simulink model "ofdm_txrx_supermimo_coded"  (Simulink 7.1)
settings: Solver=VariableStepDiscrete  SolverMode=SingleTasking  StartTime=0.0  StopTime=2*simOnly_simLength  RelTol=1e-3
PostLoadFcn:
  | ofdm_rx_supermimo_init
InitFcn:
  | ofdm_rx_supermimo_init
StartFcn:
  | ofdm_rx_supermimo_init
values from neighbouring files (symbols this model uses):
  Action0_Config = 0* 31*2^18 + 0*2^24 + PHY_AUTORESPONSE_REQ_GOODHDR + PHY_AUTORESPONSE_REQ_GOODPKT + PHY_AUTORESPONSE_REQ_FLAGA    [ofdm_tx_supermimo_init.m]
  Action1_Config = 0* 62*2^18 + PHY_AUTORESPONSE_REQ_GOODHDR + PHY_AUTORESPONSE_REQ_GOODPKT    [ofdm_tx_supermimo_init.m]
  Action2_Config = 0    [ofdm_tx_supermimo_init.m]
  Action3_Config = 0    [ofdm_tx_supermimo_init.m]
  Action4_Config = 0    [ofdm_tx_supermimo_init.m]
  Action5_Config = 0    [ofdm_tx_supermimo_init.m]
  ActionID_AFTransmit = 31    [ofdm_tx_supermimo_init.m]
  ActionID_Disabled = 0    [ofdm_tx_supermimo_init.m]
  ActionID_SetFlagA = 62    [ofdm_tx_supermimo_init.m]
  ActionID_SetFlagB = 61    [ofdm_tx_supermimo_init.m]
  BER_RAM_init_size = 2048    [ofdm_tx_supermimo_init.m]
  channel_h = [1 0; 1 0] * 0.3    [ofdm_tx_supermimo_init.m]
  CPLength = 16    [ofdm_tx_supermimo_init.m]
  FEC_Config = fec_coding_en*1 + fec_soft_dec*2 + fec_qpsk_scl*2^4 + fec_16qam_scl*2^8    (= 4195)    [ofdm_tx_supermimo_init.m]
  longCorr_coef_i = [-4*real(LTS) 0]    [ofdm_rx_supermimo_init.m]
  longCorr_coef_q = [4*imag(LTS) 0]    [ofdm_rx_supermimo_init.m]
  Match0_Config = 0    [ofdm_tx_supermimo_init.m]
  Match1_Config = 0    [ofdm_tx_supermimo_init.m]
  Match2_Config = 0    [ofdm_tx_supermimo_init.m]
  Match3_Config = 0    [ofdm_tx_supermimo_init.m]
  Match4_Config = 0    [ofdm_tx_supermimo_init.m]
  Match5_Config = 0    [ofdm_tx_supermimo_init.m]
  Match6_Config = 0    [ofdm_tx_supermimo_init.m]
  Match7_Config = 0    [ofdm_tx_supermimo_init.m]
  max_CP_length = 16    [ofdm_tx_supermimo_init.m]
  max_num_baseRateSymbols = 31    [ofdm_tx_supermimo_init.m]
  max_num_subcarriers = 64    [ofdm_tx_supermimo_init.m]
  max_num_trainingSymbols = 15    [ofdm_tx_supermimo_init.m]
  max_numBytes = 16384    [ofdm_tx_supermimo_init.m]
  max_OFDM_symbols = 1023    [ofdm_tx_supermimo_init.m]
  modConstellation_qam16 = .75*[1 3 -1 -3]./3    [ofdm_tx_supermimo_init.m]
  modConstellation_qam64 = 0.875*[3 1 5 7 -3 -1 -5 -7]./7    [ofdm_tx_supermimo_init.m]
  modConstellation_qpsk = [1 -1]./sqrt(2)    [ofdm_tx_supermimo_init.m]
  numBaseRateSymbols = numBaseRateSymbols *2    [ofdm_tx_supermimo_init.m]
  numSubcarriers = 64    [ofdm_tx_supermimo_init.m]
  numTrainingSymbols = 2    [ofdm_tx_supermimo_init.m]
  pilot_indicies = 7 + ( (64-21) * 2^8) + ( (64-7) * 2^16) + (21 * 2^24)    (= 356068103)    [ofdm_tx_supermimo_init.m]
  pilotValues = hex2dec('A57D') + (2^16 * hex2dec('5A82'))    [ofdm_tx_supermimo_init.m]
  pktByteNums = numHeaderBytes +  				byteIndex_numPayloadBytes(1)*2^8 +  				byteIndex_numPayloadBytes(2)*2^16 +  				byteIndex_simpleDynModMasks * 2^24    [ofdm_rx_supermimo_init.m]
  PktDet_Delay = 58+32 +      Symbol_Timing_Offset*2^7 +      0*2^16 +      (117* 2^24)    (= 1962935642)    [ofdm_rx_supermimo_init.m]
  pktTiming_count_payloadStart = pktTiming_count_lsStart + pktTiming_ls_num*pktTiming_ls_length    [ofdm_rx_supermimo_init.m]
  preamble = preamble_scale*[shortsyms_10 longsyms_2]    [ofdm_tx_supermimo_init.m]
  preamble_ROM = preamble_I_ROM + preamble_Q_ROM*2^16    [ofdm_tx_supermimo_init.m]
  RAM_init_size = max_numBytes    (= 16384)    [ofdm_tx_supermimo_init.m]
  reg_AF_Tx_Blanking = (320+80) + ( (320+80+79)*2^16)    (= 31392144)    [ofdm_rx_supermimo_init.m]
  reg_AF_Tx_Scaling = 2944    [ofdm_rx_supermimo_init.m]
  reg_Interrupt_PktBuf_Control = reg_InterruptControl +  	1 * 2^16 +  	0 * 2^24    (= 65536)    [ofdm_rx_supermimo_init.m]
  reg_PilotCalcParams = (4 * 2^16) + (minPilotChanMag)    (= 262184.96)    [ofdm_rx_supermimo_init.m]
  regRx_chanEst_minMag = ((0.5)*2^15) + ((0.5 * 2^15)*2^16)    (= 1073758208)    [ofdm_rx_supermimo_init.m]
  regRx_coarseCalcCorrection = round((1.3e-3 - 1.2982e-3)*2^32)    [ofdm_rx_supermimo_init.m]
  regRx_pilotCalcCorrection = round(1.00115 * 2^31)    [ofdm_rx_supermimo_init.m]
  regRx_preCFOoptions = 0 * 2^0 +  	1 * 2^1 +  	0    (= 2)    [ofdm_rx_supermimo_init.m]
  rx_controlBits = 1 * 2  		 + 1 * 4  		 + 0 * 8  		 + 1 * 16 * rx_SISO_Mode  		 + 1 * 32  		 + 1 * 64  		 + 1 * 128  		 + 1 * 256  		 + 1 * 512  		 + 1 * 1024  		 + 1 * 4096  		 + 0 * 16384  		 + 0 * 32768  		 + 1 * 65536  		 + 0 * 131072  		 + 0 * 262144  		 + 1 * 524288  		 + 1 * 1048576 * tx_Alamouti_Mode  		 + 0 * 2097152           + 1 * 33554432  		 + 0 * 4194304  		 + 0 * 8388608  		 + 0 * 16777216  		 + 1 *  ...(+49 chars)    [ofdm_rx_supermimo_init.m]
  Rx_PktDet_LongCorr_Params = LongCorrCounterLoad + 0*2^8 + 0*2^16 + 255*2^24    (= 4278190325)    [ofdm_rx_supermimo_init.m]
  Rx_PktDet_LongCorrThresholds = 8000    [ofdm_rx_supermimo_init.m]
  rx_postEq_scaling = round(rxScaling*2^11) * (1 + 2^16)    [ofdm_rx_supermimo_init.m]
  RxDataScrambling_Seq = [40 198 78 63 82 173 102 245 48 111 172 115 147 230 216 93 72 65 62 2 205 242 122 90 128 83 105 97 73 10 5 252]    [ofdm_tx_supermimo_init.m]
  RxReg_FixedPktLen = 0    [ofdm_rx_supermimo_init.m]
  rxReg_pktDetCorr = 2^00 * (floor(0.7*128)) +  	2^08 * (300) +  	0    [ofdm_rx_supermimo_init.m]
  shortSymbol_time = shortSymbol_time(1:16).'    [ofdm_tx_supermimo_init.m]
  simOnly_numSamples = length(preamble)+( (numSubcarriers+CPLength)*(numTrainingSymbols + numBaseRateSymbols + numFullRateSymbols/2) )    [ofdm_tx_supermimo_init.m]
  train = [train train]    [ofdm_tx_supermimo_init.m]
  tx_controlBits = (antB_preambleShift * 2^4) + (2^1 * tx_Alamouti_Mode) +  	(2^0 * tx_SISO_Mode) + 4*0 + 8*1 + 0*256 + 512*0 + 1024*0 +  	0*hex2dec('1000') + 0*hex2dec('2000') + 0*hex2dec('4000') +  	1*hex2dec('8000') + 1*hex2dec('10000') + hex2dec('20000') +  	0*hex2dec('100000')    [ofdm_tx_supermimo_init.m]
  tx_delays = (50*2^0) + (0*2^8) + (30*2^12)    (= 122930)    [ofdm_tx_supermimo_init.m]
  tx_scaling = (3072) + (12288 * 2^16)    (= 805309440)    [ofdm_tx_supermimo_init.m]
  TxDataScrambling_Seq = [40 198 78 63 82 173 102 245 48 111 172 115 147 230 216 93 72 65 62 2 205 242 122 90 128 83 105 97 73 10 5 252]    [ofdm_tx_supermimo_init.m]
  txReg_symbolCounts = (2^8 *numBaseRateSymbols ) + numTrainingSymbols    [ofdm_tx_supermimo_init.m]
  TxRx_FFTScaling = (3 * 2^0) +  	(2 * 2^2) +  	(1 * 2^4) +  	(1 * 2^6) +  	(1 * 2^8) +  	(0 * 2^10)    (= 347)    [ofdm_tx_supermimo_init.m]
undefined symbols (not defined in the file or in its folder): AntA, AntB, BaseRate, Down, gcbh, numBuffs, numBytes, Up, xlBlockLoadCallback, xlGetSimulinkPeriod, xlipgetpartsetting
libraries: xbsIndex_r4 x6000, simulink x4, dspsigops x4, commchan3 x2, dspsrcs4 x2, commrflib2 x2
(part 13 of 63)

== system ofdm_txrx_supermimo_coded/OFDM Rx MIMO/Coarse Freq Correction/A*B ==
  Re[A] : Inport  OutDataType=sfix(16)  OutScaling=2^0
  Im[A] : Inport  Port=2  OutDataType=sfix(16)  OutScaling=2^0
  Im[B] : Inport  Port=3  OutDataType=sfix(16)  OutScaling=2^0
  Re[B] : Inport  Port=4  OutDataType=sfix(16)  OutScaling=2^0
  Add1 : xbsIndex_r4/AddSub  mode=Addition  use_carryin=off  use_carryout=off  en=off  latency=0  precision=User Defined  arith_type="Signed  (2's comp)"  n_bits=17  bin_pt=15  quantization=Truncate  overflow=Flag as error  dbl_ovrd=off  use_behavioral_HDL=on  pipelined=off  use_rpm=on  hw_selection=Fabric  block_type=addsub
      settings: xl_area=[9 0 0 16 0 0 0]  block_version=VER_STRING_GOES_HERE
  Counter : xbsIndex_r4/Counter  Orientation=down  cnt_type=Free Running  cnt_to=4  operation=Up  start_count=0  cnt_by_val=1  arith_type=Unsigned  n_bits=2  bin_pt=0  load_pin=off  rst=off  en=off  explicit_period=on  period=1  dbl_ovrd=off  use_behavioral_HDL=off  use_rpm=on  implementation=Fabric  block_type=counter
      settings: xl_area=[3 3 0 4 0 0 0]  block_version=VER_STRING_GOES_HERE
  Down Sample3 : xbsIndex_r4/Down Sample  sample_ratio=4  sample_phase=First Value of Frame  en=off  latency=1  dbl_ovrd=off  block_type=dsamp
      settings: xl_area=[9 17 0 0 0 0 0]  block_version=VER_STRING_GOES_HERE
  Down Sample4 : xbsIndex_r4/Down Sample  sample_ratio=4  sample_phase=First Value of Frame  en=off  latency=1  dbl_ovrd=off  block_type=dsamp
      settings: xl_area=[9 17 0 0 0 0 0]  block_version=VER_STRING_GOES_HERE
  Mult4 : xbsIndex_r4/Mult  precision=User Defined  arith_type="Signed  (2's comp)"  n_bits=17  bin_pt=16  quantization=Truncate  overflow=Flag as error  en=off  latency=1  dbl_ovrd=off  use_behavioral_HDL=off  use_embedded=on  opt=Speed  optimum_pipeline=off  pipeline=off  use_rpm=on  placement_style=Rectangular shape  block_type=mult
      settings: infoedit=Hardware notes: To use the internal pipeline stage of the dedicated multiplier you must select 'Pipeline for maximum performance'.  xl_area=[14 27 0 27 0 1 0]  block_version=VER_STRING_GOES_HERE
  Mux : xbsIndex_r4/Mux  inputs=4  en=off  latency=0  precision=Full  arith_type="Signed  (2's comp)"  n_bits=16  bin_pt=13  quantization=Truncate  overflow=Wrap  dbl_ovrd=off  block_type=mux
      settings: xl_area=[14 0 0 28 0 0 0]  block_version=VER_STRING_GOES_HERE
  Mux1 : xbsIndex_r4/Mux  inputs=4  en=off  latency=0  precision=Full  arith_type="Signed  (2's comp)"  n_bits=16  bin_pt=13  quantization=Truncate  overflow=Wrap  dbl_ovrd=off  block_type=mux
      settings: xl_area=[14 0 0 28 0 0 0]  block_version=VER_STRING_GOES_HERE
  Negate : xbsIndex_r4/Negate  precision=Full  arith_type="Signed  (2's comp)"  n_bits=14  bin_pt=13  quantization=Truncate  overflow=Flag as error  en=off  latency=0  dbl_ovrd=off  block_type=negate
      settings: xl_area=[7 0 0 14 0 0 0]  block_version=VER_STRING_GOES_HERE
  Register : xbsIndex_r4/Register  init=0  rst=off  en=off  dbl_ovrd=off  block_type=register
      settings: block_version=10.1.2
  Register1 : xbsIndex_r4/Register  init=0  rst=off  en=off  dbl_ovrd=off  block_type=register
      settings: block_version=10.1.2
  Register2 : xbsIndex_r4/Register  init=0  rst=off  en=off  dbl_ovrd=off  block_type=register
      settings: block_version=10.1.2
  Slice : xbsIndex_r4/Slice  nbits=2  boolean_output=off  mode=Lower Bit Location + Width  bit1=0  base1=MSB of Input  bit0=0  base0=LSB of Input  dbl_ovrd=off  block_type=slice
      settings: infoedit="Extracts a given range of bits from each input sample and presents it at the output.  The output type is ordinarily unsigned with binary point at zero, but can be Boolean when the slice is one bit wide.<P><P>Hardware notes: In hardware this block costs nothing."  block_version=VER_STRING_GOES_HERE
  Up Sample{1..4} (4 alike) : xbsIndex_r4/Up Sample  sample_ratio=4  copy_samples=on  dbl_ovrd=off  block_type=usamp
      settings: infoedit="Up samples input data.  Inserted values  can be zeros or copies of the most recent input sample.<P><P>Hardware notes: No hardware is needed if inserted values are copies of the input sample; otherwise, a mux and single bit flip-flop are used."  xl_area=[0 0 0 0 0 0 0]  block_version=VER_STRING_GOES_HERE
  Re : Outport  OutDataType=sfix(16)  OutScaling=2^0
  Im1 : Outport  Port=2  OutDataType=sfix(16)  OutScaling=2^0
  wires:
  Slice/1 -> Mux/1, Mux1/1
  Counter/1 -> Slice/1
  Register2/1 -> Add1/2
  Mult4/1 -> Add1/1, Register2/1
  Up Sample1/1 -> Mux1/5, Negate/1
  Up Sample4/1 -> Mux/4, Mux/3
  Up Sample2/1 -> Mux/2, Mux/5
  Up Sample3/1 -> Mux1/4, Mux1/2
  Re[A]/1 -> Up Sample3/1
  Re[B]/1 -> Up Sample2/1
  Im[A]/1 -> Up Sample1/1
  Im[B]/1 -> Up Sample4/1
  Mux1/1 -> Mult4/1
  Mux/1 -> Mult4/2
  Register/1 -> Register1/1
  Down Sample4/1 -> Im1/1
  Down Sample3/1 -> Re/1
  Add1/1 -> Down Sample4/1, Register/1
  Negate/1 -> Mux1/3
  Register1/1 -> Down Sample3/1

== system ofdm_txrx_supermimo_coded/OFDM Rx MIMO/Coarse Freq Correction/A*B1 ==
  Re[B] : Inport  OutDataType=sfix(16)  OutScaling=2^0
  Im[B] : Inport  Port=2  OutDataType=sfix(16)  OutScaling=2^0
  Re[A] : Inport  Port=3  OutDataType=sfix(16)  OutScaling=2^0
  Im[A] : Inport  Port=4  OutDataType=sfix(16)  OutScaling=2^0
  Add1 : xbsIndex_r4/AddSub  mode=Addition  use_carryin=off  use_carryout=off  en=off  latency=0  precision=User Defined  arith_type="Signed  (2's comp)"  n_bits=17  bin_pt=15  quantization=Truncate  overflow=Flag as error  dbl_ovrd=off  use_behavioral_HDL=on  pipelined=off  use_rpm=on  hw_selection=Fabric  block_type=addsub
      settings: xl_area=[9 0 0 16 0 0 0]  block_version=VER_STRING_GOES_HERE
  Counter : xbsIndex_r4/Counter  Orientation=down  cnt_type=Free Running  cnt_to=4  operation=Up  start_count=0  cnt_by_val=1  arith_type=Unsigned  n_bits=2  bin_pt=0  load_pin=off  rst=off  en=off  explicit_period=on  period=1  dbl_ovrd=off  use_behavioral_HDL=off  use_rpm=on  implementation=Fabric  block_type=counter
      settings: xl_area=[3 3 0 4 0 0 0]  block_version=VER_STRING_GOES_HERE
  Delay1 : xbsIndex_r4/Delay  en=off  latency=1  dbl_ovrd=off  reg_retiming=off  block_type=delay
      settings: infoedit=Hardware notes: A delay line is a chain, each link of which is an SRL16 followed by a flip-flop. If register retiming is enabled, the delay line is a chain of flip-flops.  xl_area=[8 16 0 0 0 0 0]  block_version=VER_STRING_GOES_HERE
  Delay2 : xbsIndex_r4/Delay  en=off  latency=2  dbl_ovrd=off  reg_retiming=off  block_type=delay
      settings: infoedit=Hardware notes: A delay line is a chain, each link of which is an SRL16 followed by a flip-flop. If register retiming is enabled, the delay line is a chain of flip-flops.  xl_area=[9 17 0 17 0 0 0]  block_version=VER_STRING_GOES_HERE
  Down Sample3 : xbsIndex_r4/Down Sample  sample_ratio=4  sample_phase=First Value of Frame  en=off  latency=1  dbl_ovrd=off  block_type=dsamp
      settings: xl_area=[9 17 0 0 0 0 0]  block_version=VER_STRING_GOES_HERE
  Down Sample4 : xbsIndex_r4/Down Sample  sample_ratio=4  sample_phase=First Value of Frame  en=off  latency=1  dbl_ovrd=off  block_type=dsamp
      settings: xl_area=[9 17 0 0 0 0 0]  block_version=VER_STRING_GOES_HERE
  Mult4 : xbsIndex_r4/Mult  precision=User Defined  arith_type="Signed  (2's comp)"  n_bits=16  bin_pt=15  quantization=Truncate  overflow=Flag as error  en=off  latency=1  dbl_ovrd=off  use_behavioral_HDL=off  use_embedded=on  opt=Speed  optimum_pipeline=off  pipeline=off  use_rpm=on  placement_style=Rectangular shape  block_type=mult
      settings: infoedit=Hardware notes: To use the internal pipeline stage of the dedicated multiplier you must select 'Pipeline for maximum performance'.  xl_area=[14 27 0 27 0 1 0]  block_version=VER_STRING_GOES_HERE
  Mux : xbsIndex_r4/Mux  inputs=4  en=off  latency=0  precision=Full  arith_type="Signed  (2's comp)"  n_bits=16  bin_pt=13  quantization=Truncate  overflow=Wrap  dbl_ovrd=off  block_type=mux
      settings: xl_area=[14 0 0 28 0 0 0]  block_version=VER_STRING_GOES_HERE
  Mux1 : xbsIndex_r4/Mux  inputs=4  en=off  latency=0  precision=Full  arith_type="Signed  (2's comp)"  n_bits=16  bin_pt=13  quantization=Truncate  overflow=Wrap  dbl_ovrd=off  block_type=mux
      settings: xl_area=[14 0 0 28 0 0 0]  block_version=VER_STRING_GOES_HERE
  Negate : xbsIndex_r4/Negate  precision=Full  arith_type="Signed  (2's comp)"  n_bits=32  bin_pt=30  quantization=Truncate  overflow=Flag as error  en=off  latency=0  dbl_ovrd=off  block_type=negate
      settings: xl_area=[7 0 0 14 0 0 0]  block_version=VER_STRING_GOES_HERE
  Slice : xbsIndex_r4/Slice  nbits=2  boolean_output=off  mode=Lower Bit Location + Width  bit1=0  base1=MSB of Input  bit0=0  base0=LSB of Input  dbl_ovrd=off  block_type=slice
      settings: infoedit="Extracts a given range of bits from each input sample and presents it at the output.  The output type is ordinarily unsigned with binary point at zero, but can be Boolean when the slice is one bit wide.<P><P>Hardware notes: In hardware this block costs nothing."  block_version=VER_STRING_GOES_HERE
  Up Sample{1..4} (4 alike) : xbsIndex_r4/Up Sample  sample_ratio=4  copy_samples=on  dbl_ovrd=off  block_type=usamp
      settings: infoedit="Up samples input data.  Inserted values  can be zeros or copies of the most recent input sample.<P><P>Hardware notes: No hardware is needed if inserted values are copies of the input sample; otherwise, a mux and single bit flip-flop are used."  xl_area=[0 0 0 0 0 0 0]  block_version=VER_STRING_GOES_HERE
  Re : Outport  OutDataType=sfix(16)  OutScaling=2^0
  Im1 : Outport  Port=2  OutDataType=sfix(16)  OutScaling=2^0
  wires:
  Add1/1 -> Delay2/1, Down Sample4/1
  Down Sample3/1 -> Re/1
  Down Sample4/1 -> Im1/1
  Delay2/1 -> Down Sample3/1
  Mux/1 -> Mult4/2
  Mux1/1 -> Mult4/1
  Im[B]/1 -> Up Sample4/1
  Im[A]/1 -> Up Sample1/1
  Re[B]/1 -> Up Sample2/1
  Re[A]/1 -> Up Sample3/1
  Up Sample3/1 -> Mux1/2, Mux1/4
  Up Sample2/1 -> Mux/5, Mux/2
  Up Sample4/1 -> Mux/3, Mux/4
  Up Sample1/1 -> Mux1/5, Negate/1
  Mult4/1 -> Add1/1, Delay1/1
  Delay1/1 -> Add1/2
  Counter/1 -> Slice/1
  Slice/1 -> Mux1/1, Mux/1
  Negate/1 -> Mux1/3

== system ofdm_txrx_supermimo_coded/OFDM Rx MIMO/Coarse Freq Correction/Coarse CFO Calc ==
  note: Running sum of last 64 samples
      | Full-precision avoids overflow, which would
      | totally screw up this recursive calculation.
  note: (1/64) for avgLen
      | (1/64) for time_diff
  I In : Inport  OutDataType=sfix(16)  OutScaling=2^0
  Q In : Inport  Port=2  OutDataType=sfix(16)  OutScaling=2^0
  RxPkt Active : Inport  Port=3  OutDataType=sfix(16)  OutScaling=2^0
  Reset : Inport  Port=4  OutDataType=sfix(16)  OutScaling=2^0
  ASR : xbsIndex_r4/Addressable Shift Register  infer_latency=on  depth=2  initVector=[0]  en=off  dbl_ovrd=off  use_behavioral_HDL=off  use_rpm=off  block_type=addrsr
      settings: block_version=8.2.02
      settings: infoedit=|
      | Delay of  configurable length.  Any element  in the delay
      | 
      |     line   can    be   addressed   and   driven    onto   the   output
      | 
      |     port.<P><P>Hardware  notes: Implemented  using SRL16s.
      | 
      |     If Virtex-4, Virtex-II or Spartan-3 devices are used, multiple SRLC16s are cascaded together.
  Accum : xbsIndex_r4/Accumulator  operation=Add  n_bits=24+6+6  overflow=Flag as error  scale=1  rst=off  hasbypass=on  en=off  dbl_ovrd=off  use_behavioral_HDL=on  implementation=Fabric  block_type=accum
      settings: infoedit="Adder or subtractor-based accumulator.   Output type and binary point position match the input.<P><P>Hardware notes: When \"Reinitialize with input 'b' on reset\" is selected, the accumulator is forced to run at the system rate even if the input 'b' is running at a slower rate."  xl_area=[17 33 0 32 0 0 0]  block_version=VER_STRING_GOES_HERE
  AddSub1 : xbsIndex_r4/AddSub  mode=Subtraction  use_carryin=off  use_carryout=off  en=off  latency=0  precision=Full  arith_type="Signed  (2's comp)"  n_bits=24  bin_pt=24  quantization="Round  (unbiased: +/- Inf)"  overflow=Wrap  dbl_ovrd=off  use_behavioral_HDL=off  pipelined=off  use_rpm=on  hw_selection=Fabric  block_type=addsub
      settings: block_version=VER_STRING_GOES_HERE
  AddSub2 : xbsIndex_r4/AddSub  mode=Addition  use_carryin=off  use_carryout=off  en=off  latency=0  precision=User Defined  arith_type="Signed  (2's comp)"  n_bits=32  bin_pt=32  quantization="Round  (unbiased: +/- Inf)"  overflow=Wrap  dbl_ovrd=off  use_behavioral_HDL=off  pipelined=off  use_rpm=on  hw_selection=Fabric  block_type=addsub
      settings: block_version=VER_STRING_GOES_HERE
  Arctan2 : Subsystem
  CFO : Scope  NumInputPorts=7
      settings: TimeRange=392.7801724137931  YMin=-0.5~-0.25~-1~-1~0~0~0  YMax=0.5~0.15~1~1~1~3500~1  SaveName=ScopeData17
  CFO1 : Scope  NumInputPorts=5
      settings: TimeRange=14608  YMin=-0.25~-0.25~-1~-1~0  YMax=0.2~0.25~1~1~1  SaveName=ScopeData27
  Convert1 : xbsIndex_r4/Convert  arith_type=Unsigned  n_bits=32  bin_pt=32  quantization=Truncate  overflow=Wrap  latency=0  dbl_ovrd=off  pipeline=off  block_type=convert
      settings: xl_area=[0 0 0 0 0 0 0]  block_version=VER_STRING_GOES_HERE
  Delay1 : xbsIndex_r4/Delay  en=off  latency=64  dbl_ovrd=off  reg_retiming=off  block_type=delay
      settings: infoedit=Hardware notes: A delay line is a chain, each link of which is an SRL16 followed by a flip-flop. If register retiming is enabled, the delay line is a chain of flip-flops.  block_version=VER_STRING_GOES_HERE
  Delay2 : xbsIndex_r4/Delay  en=off  latency=1  dbl_ovrd=off  reg_retiming=off  block_type=delay
      settings: infoedit=Hardware notes: A delay line is a chain, each link of which is an SRL16 followed by a flip-flop. If register retiming is enabled, the delay line is a chain of flip-flops.  block_version=VER_STRING_GOES_HERE
  Down Sample2 : xbsIndex_r4/Down Sample  sample_ratio=4  sample_phase="Last Value of Frame  (most efficient)"  en=off  latency=1  dbl_ovrd=off  block_type=dsamp
      settings: block_version=VER_STRING_GOES_HERE
  Gateway Out{1..5} (5 alike) : xbsIndex_r4/Gateway Out  hdl_port=off  timing_constraint=None  locs_specified=off  LOCs={}  block_type=gatewayout
      settings: infoedit="Gateway out block.  Converts Xilinx fixed point inputs into ouputs of type Simulink integer, double, or fixed point.<P><P>Hardware notes:  In hardware these blocks become top level output ports or are discarded, depending on how they are configured."  xl_area=[0 0 0 0 0 0 0]  block_version=VER_STRING_GOES_HERE
  Inverter1 : xbsIndex_r4/Inverter  en=off  latency=0  dbl_ovrd=off  block_type=inv
      settings: xl_area=[0 0 0 0 0 0 0]  block_version=VER_STRING_GOES_HERE
  Inverter2 : xbsIndex_r4/Inverter  en=off  latency=0  dbl_ovrd=off  block_type=inv
      settings: xl_area=[0 0 0 0 0 0 0]  block_version=VER_STRING_GOES_HERE
  Logical : xbsIndex_r4/Logical  logical_function=AND  inputs=2  en=off  latency=0  precision=Full  arith_type=Unsigned  n_bits=16  bin_pt=0  align_bp=on  dbl_ovrd=off  block_type=logical
      settings: block_version=8.2.01
  Logical1 : xbsIndex_r4/Logical  logical_function=OR  inputs=3  en=off  latency=0  precision=Full  arith_type=Unsigned  n_bits=16  bin_pt=0  align_bp=on  dbl_ovrd=off  block_type=logical
      settings: block_version=8.2.01
  Logical2 : xbsIndex_r4/Logical  logical_function=OR  inputs=2  en=off  latency=0  precision=Full  arith_type=Unsigned  n_bits=16  bin_pt=0  align_bp=on  dbl_ovrd=off  block_type=logical
      settings: block_version=8.2.01
  PhaseDiff : Subsystem
  PktDet-Only CoarseCFO Timing : Subsystem
  Posedge3 : Subsystem
  Register4 : xbsIndex_r4/Register  init=0  rst=on  en=on  dbl_ovrd=off  block_type=register
      settings: block_version=8.2.01
  S-R Latch1 : Subsystem
  Scale : xbsIndex_r4/Scale  scale_factor=-12  dbl_ovrd=off  block_type=scale
      settings: infoedit=Scales input by a power of two by adjusting the binary point position.<P><P>Hardware notes: In hardware this block costs nothing.  block_version=10.1.2
  Phase Inc : Outport  OutDataType=sfix(16)  OutScaling=2^0
  tag scope: preSpin_coarseRxCFOest=global, preSpin_coarseRxCFOest_valid=global, regRx_coarseCFOest=global, regRx_coarseCFOest_en=global
  wires:
  I In/1 -> Arctan2/1(I)
  Q In/1 -> Arctan2/2(Q)
  Arctan2/1(atan) -> PhaseDiff/1(p), Gateway Out3/1, CFO/3
  PhaseDiff/1(diff(p0,p63)) -> AddSub1/1, Delay1/1, Gateway Out2/1
  Delay1/1 -> AddSub1/2
  AddSub1/1 -> Accum/1, Gateway Out1/1
  Accum/1 -> Gateway Out4/1, Register4/1
  AddSub2/1 -> Convert1/1, Gateway Out5/1
  Logical2/1 -> ASR/1
  [regRx_CFOCalc_Dly] -> ASR/2
  ASR/1 -> Logical/2
  Inverter2/1 -> Logical1/3
  Posedge3/1(Q) -> Register4/3, Delay2/1
  Logical1/1 -> Register4/2, S-R Latch1/1(R)
  RxPkt Active/1 -> Inverter2/1, Logical/1
  Reset/1 -> Logical1/1
  [regRx_coarseCFO_en] -> Inverter1/1
  Inverter1/1 -> Logical1/2
  Register4/1 -> Scale/1
  Logical/1 -> S-R Latch1/2(S)
  S-R Latch1/1(Q) -> Posedge3/1(D)
  Delay2/1 -> [regRx_coarseCFOest_en], [preSpin_coarseRxCFOest_valid]
  Scale/1 -> AddSub2/2
  Gateway Out2/1 -> CFO1/2
  Gateway Out3/1 -> CFO1/1
  Gateway Out1/1 -> CFO1/3
  Gateway Out4/1 -> CFO1/4
  Gateway Out5/1 -> CFO1/5
  [regRx_coarseCFO_correction] -> Down Sample2/1
  Convert1/1 -> [regRx_coarseCFOest], [preSpin_coarseRxCFOest], Phase Inc/1
  Down Sample2/1 -> AddSub2/1
  [longCorrAboveThresh_Twice] -> Logical2/1, CFO/1
  PktDet-Only CoarseCFO Timing/1(Capt) -> Logical2/2
  [RxPktTiming_SampleCount] -> CFO/2

== system ofdm_txrx_supermimo_coded/OFDM Rx MIMO/Coarse Freq Correction/DDS ==
  Tx_PhaseInc : Inport  OutDataType=sfix(16)  OutScaling=2^0
  Rx_CoarseEst : Inport  Port=2  OutDataType=sfix(16)  OutScaling=2^0
  Rst : Inport  Port=3  OutDataType=sfix(16)  OutScaling=2^0
  "DDS Compiler 2.1 " : xbsIndex_r4/DDS Compiler 2.1   output_selection=Sine_and_Cosine  negative_sine=on  negative_cosine=off  channels=1  dds_clock_rate=40  spurious_free_dynamic_range=106  frequency_resolution=0.4  rst=on  en=off  rfd=off  rdy=off  channel_pin=off  explicit_period=off  period=1  phase_increment=Programmable  output_frequency1=0  output_frequency2=0  output_frequency3=0  output_frequency4=0  output_frequency5=0  output_frequency6=0  output_frequency7=0  output_frequency8=0  output_frequency9=0  output_frequency10=0  output_frequency11=0  output_frequency12=0  output_frequency13=0  output_frequency14=0  output_frequency15=0  output_frequency16=0  phase_offset=None  phase_offset_angles1=0  phase_offset_angles2=0  phase_offset_angles3=0  phase_offset_angles4=0  phase_offset_angles5=0  phase_offset_angles6=0  phase_offset_angles7=0  phase_offset_angles8=0  phase_offset_angles9=0  phase_offset_angles10=0  phase_offset_angles11=0  phase_offset_angles12=0  phase_offset_angles13=0  phase_offset_angles14=0  phase_offset_angles15=0  phase_offset_angles16=0  memory_type=Auto  optimization_goal=Auto  dsp48_use=Minimal  noise_shaping=Auto  latency_configuration=Auto  latency=1  accumulator_latency=One  por_mode=false  ip_name=DDS Compiler  ip_version=2.1  dsptool_ready=true  wrapper_available=true  structural_sim=false  clock_enable=true  sclr_pin=false  port_translation_map={ 'ce' => 'en', 'sclr' => 'rst'}  block_type=dds_compiler_v2_1
      settings: block_version=10.1.3
  Indeterminate Checker : Subsystem
  Phase Inc Sel : Subsystem
  inv 2pi2 : xbsIndex_r4/Constant  arith_type=Boolean  const=1  n_bits=32  bin_pt=32  explicit_period=off  period=1  equ=P=C  opselect=C  inp2=PCIN>>17  opr=+  inp1=P  carry=CIN  dbl_ovrd=off  block_type=constant
      settings: block_version=VER_STRING_GOES_HERE
  Q : Outport  OutDataType=sfix(16)  OutScaling=2^0
  I : Outport  Port=2  OutDataType=sfix(16)  OutScaling=2^0
  tag scope: CFO_DDSOut_I=global, CFO_DDSOut_Q=global
  wires:
  inv 2pi2/1 -> "DDS Compiler 2.1 "/1
  Phase Inc Sel/1(DDS_PhaseInc) -> "DDS Compiler 2.1 "/2
  "DDS Compiler 2.1 "/1 -> Indeterminate Checker/1(-sin)
  "DDS Compiler 2.1 "/2 -> Indeterminate Checker/2(cos)
  Indeterminate Checker/1(" -sin") -> Q/1, [CFO_DDSOut_Q]
  Indeterminate Checker/2(" cos") -> I/1, [CFO_DDSOut_I]
  Phase Inc Sel/2(DDS_Rst) -> "DDS Compiler 2.1 "/3
  Rx_CoarseEst/1 -> Phase Inc Sel/1(Rx_CoarseEst)
  Rst/1 -> Phase Inc Sel/2(Rst)
  Tx_PhaseInc/1 -> Phase Inc Sel/3(Tx_PhaseInc)

== system ofdm_txrx_supermimo_coded/OFDM Rx MIMO/Coarse Freq Correction/PreSpin CFO Sel ==
  note: If:
      | UsePilots and UseCoarse, capture after pilots are valid
      | UsePilots but NOT UseCoarse, capture after pilots are valid
      | NOT UsePilots but UseCoarse, capture after coarse is valid
      | If neither, don't capture
  note: Safety check: never capture when Tx is running
      | (shouldn't happen)
  Rst : Inport
  5LSB+8 : xbsIndex_r4/Slice  nbits=5  boolean_output=off  mode=Lower Bit Location + Width  bit1=0  base1=MSB of Input  bit0=8  base0=LSB of Input  dbl_ovrd=off  block_type=slice
      settings: infoedit="Extracts a given range of bits from each input sample and presents it at the output.  The output type is ordinarily unsigned with binary point at zero, but can be Boolean when the slice is one bit wide.<P><P>Hardware notes: In hardware this block costs nothing."  block_version=VER_STRING_GOES_HERE
  AddSub1 : xbsIndex_r4/AddSub  mode=Addition  use_carryin=off  use_carryout=off  en=off  latency=0  precision=User Defined  arith_type=Unsigned  n_bits=32  bin_pt=32  quantization=Truncate  overflow=Wrap  dbl_ovrd=off  use_behavioral_HDL=off  pipelined=off  use_rpm=on  hw_selection=Fabric  block_type=addsub
      settings: block_version=VER_STRING_GOES_HERE
  Constant2 : xbsIndex_r4/Constant  arith_type=Boolean  const=0  n_bits=1  bin_pt=0  explicit_period=on  period=1  equ=P=C  opselect=C  inp2=PCIN>>17  opr=+  inp1=P  carry=CIN  dbl_ovrd=off  block_type=constant
      settings: block_version=8.2.02
  Convert2 : xbsIndex_r4/Convert  arith_type=Boolean  n_bits=16  bin_pt=15  quantization=Truncate  overflow=Flag as error  latency=0  dbl_ovrd=off  pipeline=off  block_type=convert
      settings: xl_area=[0 0 0 0 0 0 0]  block_version=VER_STRING_GOES_HERE
  Convert5 : xbsIndex_r4/Convert  arith_type=Boolean  n_bits=16  bin_pt=15  quantization=Truncate  overflow=Flag as error  latency=0  dbl_ovrd=off  pipeline=off  block_type=convert
      settings: xl_area=[0 0 0 0 0 0 0]  block_version=VER_STRING_GOES_HERE
  Delay1 : xbsIndex_r4/Delay  en=off  latency=1  dbl_ovrd=off  reg_retiming=off  block_type=delay
      settings: infoedit=Hardware notes: A delay line is a chain, each link of which is an SRL16 followed by a flip-flop. If register retiming is enabled, the delay line is a chain of flip-flops.  block_version=VER_STRING_GOES_HERE
  Down Sample : xbsIndex_r4/Down Sample  sample_ratio=4  sample_phase="Last Value of Frame  (most efficient)"  en=off  latency=1  dbl_ovrd=off  block_type=dsamp
      settings: block_version=10.1.3
  Gateway Out{1..5} (5 alike) : xbsIndex_r4/Gateway Out  hdl_port=off  timing_constraint=None  locs_specified=off  LOCs={}  block_type=gatewayout
      settings: infoedit="Gateway out block.  Converts Xilinx fixed point inputs into ouputs of type Simulink integer, double, or fixed point.<P><P>Hardware notes:  In hardware these blocks become top level output ports or are discarded, depending on how they are configured."  xl_area=[0 0 0 0 0 0 0]  block_version=VER_STRING_GOES_HERE
  Inverter : xbsIndex_r4/Inverter  en=off  latency=0  dbl_ovrd=off  block_type=inv
      settings: xl_area=[0 0 0 0 0 0 0]  block_version=VER_STRING_GOES_HERE
  Inverter1 : xbsIndex_r4/Inverter  en=off  latency=0  dbl_ovrd=off  block_type=inv
      settings: xl_area=[0 0 0 0 0 0 0]  block_version=VER_STRING_GOES_HERE
  Inverter2 : xbsIndex_r4/Inverter  en=off  latency=0  dbl_ovrd=off  block_type=inv
      settings: xl_area=[0 0 0 0 0 0 0]  block_version=VER_STRING_GOES_HERE
  Logical{1..2,4..7} (6 alike) : xbsIndex_r4/Logical  logical_function=AND  inputs=2  en=off  latency=0  precision=Full  arith_type=Unsigned  n_bits=8  bin_pt=2  align_bp=on  dbl_ovrd=off  block_type=logical
      settings: xl_area=[1 0 0 1 0 0 0]  block_version=VER_STRING_GOES_HERE
  Logical3 : xbsIndex_r4/Logical  logical_function=OR  inputs=3  en=off  latency=0  precision=Full  arith_type=Unsigned  n_bits=8  bin_pt=2  align_bp=on  dbl_ovrd=off  block_type=logical
      settings: xl_area=[1 0 0 1 0 0 0]  block_version=VER_STRING_GOES_HERE
  Mux : xbsIndex_r4/Mux  inputs=2  en=off  latency=0  precision=Full  arith_type=Unsigned  n_bits=16  bin_pt=14  quantization=Truncate  overflow=Wrap  dbl_ovrd=off  block_type=mux
      settings: block_version=8.2.01
  Posedge : Subsystem
  Register1 : xbsIndex_r4/Register  init=0  rst=on  en=on  dbl_ovrd=off  block_type=register
      settings: block_version=8.2.01
  Register4 : xbsIndex_r4/Register  init=0  rst=on  en=on  dbl_ovrd=off  block_type=register
      settings: block_version=8.2.01
  Shared Memory : xbsIndex_r4/Shared Memory  shared_memory_name='PktBufFreqOffsets'  depth=32  ownership=Locally Owned and Initialized  initVector=0  mutex=Unprotected  mode=Read and Write  write_mode=Read After Write  time_out=0  latency=1  explicit_data_type=on  arith_type=Unsigned  n_bits=32  bin_pt=32  implementation=Block RAM  use_rpm=off  block_type=shmem
      settings: AttributesFormatString=<< %<shared_memory_name> >>  block_version=10.1.3
  Simulation Multiplexer1 : xbsIndex_r4/Simulation Multiplexer  sim_sel=1  hw_sel=2  block_type=simmux
      settings: infoedit="Distinguish input subsystems as \"simulation only\" and \"generation only\".  The input specified For Simulation will be used during Simulink simulation.  The input specified For Generation will be used during code generation.  This block will typically be used on the output of a HW Co-Simulation, Black Box, or ModelSim subsystem.<P><P>Hardware Notes: This block costs nothing."  block_version=10.1.3
  Up Sample{,1..4} (5 alike) : xbsIndex_r4/Up Sample  sample_ratio=4  copy_samples=on  dbl_ovrd=off  block_type=usamp
      settings: infoedit="Up samples input data.  Inserted values  can be zeros or copies of the most recent input sample.<P><P>Hardware notes: No hardware is needed if inserted values are copies of the input sample; otherwise, a mux and single bit flip-flop are used."  block_version=10.1.3
  Tx_PhaseInc : Outport
  wires:
  [preSpin_pilotCFOest] -> Register4/1
  [preSpin_pilotCFOest_valid] -> Logical1/1
  [preSpin_coarseRxCFOest] -> Up Sample1/1
  [preSpin_coarseRxCFOest_valid] -> Up Sample2/1
  Register4/1 -> AddSub1/1, Gateway Out2/1
  Register1/1 -> AddSub1/2, Gateway Out1/1
  AddSub1/1 -> Gateway Out4/1, Shared Memory/2
  [regTxRx_PktBuffOffsets] -> 5LSB+8/1
  Logical1/1 -> Register4/3, Logical4/1, Logical6/1
  Logical2/1 -> Register1/3, Logical5/1
  [RxReg_PreCFO_UseCoarse] -> Up Sample3/1
  [RxReg_PreCFO_UsePilots] -> Up Sample/1
  Logical4/1 -> Logical3/2
  Inverter/1 -> Logical4/2
  Logical3/1 -> Delay1/1
  Posedge/1(Q) -> Shared Memory/3, Gateway Out5/1
  [Tx_Running] -> Up Sample4/1
  5LSB+8/1 -> Mux/2
  [PreSpin_TxBufIndex] -> Mux/3
  Mux/1 -> Shared Memory/1, Gateway Out3/1
  Rst/1 -> Register4/2, Register1/2
  Shared Memory/1 -> Down Sample/1
  Convert2/1 -> Logical2/2, Inverter/1, Logical6/2
  Convert5/1 -> Logical1/2, Inverter1/1
  Gateway Out2/1 -> ?
  Gateway Out3/1 -> ?
  Gateway Out1/1 -> ?
  Gateway Out4/1 -> ?
  Gateway Out5/1 -> ?
  Inverter1/1 -> Logical5/2
  Logical5/1 -> Logical3/3
  Logical6/1 -> Logical3/1
  Delay1/1 -> Logical7/2
  Inverter2/1 -> Logical7/1
  Logical7/1 -> Posedge/1(D)
  Constant2/1 -> Simulation Multiplexer1/1
  Simulation Multiplexer1/1 -> Inverter2/1
  Up Sample/1 -> Convert5/1
  Up Sample1/1 -> Register1/1
  Up Sample2/1 -> Logical2/1
  Up Sample3/1 -> Convert2/1
  Up Sample4/1 -> Mux/1, Simulation Multiplexer1/2
  Down Sample/1 -> Tx_PhaseInc/1

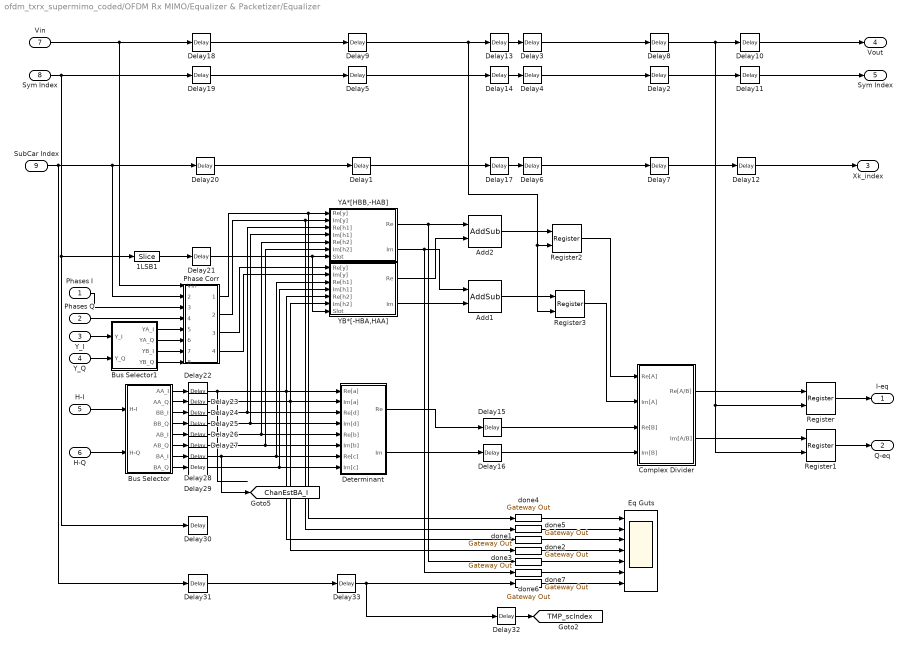
== system ofdm_txrx_supermimo_coded/OFDM Rx MIMO/Equalizer & Packetizer/Equalizer ==
  Phases I : Inport  OutDataType=sfix(16)  OutScaling=2^0
  Phases Q : Inport  Port=2  OutDataType=sfix(16)  OutScaling=2^0
  Y_I : Inport  Port=3  OutDataType=sfix(16)  OutScaling=2^0
  Y_Q : Inport  Port=4  OutDataType=sfix(16)  OutScaling=2^0
  H-I : Inport  Port=5  OutDataType=sfix(16)  OutScaling=2^0
  H-Q : Inport  Port=6  OutDataType=sfix(16)  OutScaling=2^0
  Vin : Inport  Port=7  OutDataType=sfix(16)  OutScaling=2^0
  Sym Index : Inport  Port=8  OutDataType=sfix(16)  OutScaling=2^0
  SubCar Index : Inport  Port=9  OutDataType=sfix(16)  OutScaling=2^0
  1LSB1 : xbsIndex_r4/Slice  nbits=1  boolean_output=off  mode=Lower Bit Location + Width  bit1=0  base1=MSB of Input  bit0=0  base0=LSB of Input  dbl_ovrd=off  block_type=slice
      settings: infoedit="Extracts a given range of bits from each input sample and presents it at the output.  The output type is ordinarily unsigned with binary point at zero, but can be Boolean when the slice is one bit wide.<P><P>Hardware notes: In hardware this block costs nothing."  block_version=VER_STRING_GOES_HERE
  Add1 : xbsIndex_r4/AddSub  mode=Addition  use_carryin=off  use_carryout=off  en=off  latency=0  precision=Full  arith_type="Signed  (2's comp)"  n_bits=18  bin_pt=16  quantization=Truncate  overflow=Flag as error  dbl_ovrd=off  use_behavioral_HDL=on  pipelined=off  use_rpm=on  hw_selection=Fabric  block_type=addsub
      settings: xl_area=[9 0 0 16 0 0 0]  block_version=VER_STRING_GOES_HERE
  Add2 : xbsIndex_r4/AddSub  mode=Addition  use_carryin=off  use_carryout=off  en=off  latency=0  precision=Full  arith_type="Signed  (2's comp)"  n_bits=18  bin_pt=16  quantization=Truncate  overflow=Flag as error  dbl_ovrd=off  use_behavioral_HDL=on  pipelined=off  use_rpm=on  hw_selection=Fabric  block_type=addsub
      settings: xl_area=[9 0 0 16 0 0 0]  block_version=VER_STRING_GOES_HERE
  Bus Selector : Subsystem
  Bus Selector1 : Subsystem
  Complex Divider : Subsystem
  Delay{1,3..6,9,18..31,33} (21 alike) : xbsIndex_r4/Delay  en=off  latency=2  dbl_ovrd=off  reg_retiming=off  block_type=delay
      settings: infoedit=Hardware notes: A delay line is a chain, each link of which is an SRL16 followed by a flip-flop. If register retiming is enabled, the delay line is a chain of flip-flops.  xl_area=[0, 0, 0, 0, 0, 0, 0]  block_version=VER_STRING_GOES_HERE
  Delay{10..17,32} (9 alike) : xbsIndex_r4/Delay  en=off  latency=1  dbl_ovrd=off  reg_retiming=off  block_type=delay
      settings: infoedit=Hardware notes: A delay line is a chain, each link of which is an SRL16 followed by a flip-flop. If register retiming is enabled, the delay line is a chain of flip-flops.  xl_area=[0, 0, 0, 0, 0, 0, 0]  block_version=VER_STRING_GOES_HERE
  Delay2 : xbsIndex_r4/Delay  en=off  latency=19+2+1  dbl_ovrd=off  reg_retiming=off  block_type=delay
      settings: infoedit=Hardware notes: A delay line is a chain, each link of which is an SRL16 followed by a flip-flop. If register retiming is enabled, the delay line is a chain of flip-flops.  xl_area=[0, 0, 0, 0, 0, 0, 0]  block_version=VER_STRING_GOES_HERE
  Delay7 : xbsIndex_r4/Delay  en=off  latency=19+2+1  dbl_ovrd=off  reg_retiming=off  block_type=delay
      settings: infoedit=Hardware notes: A delay line is a chain, each link of which is an SRL16 followed by a flip-flop. If register retiming is enabled, the delay line is a chain of flip-flops.  xl_area=[0, 0, 0, 0, 0, 0, 0]  block_version=VER_STRING_GOES_HERE
  Delay8 : xbsIndex_r4/Delay  en=off  latency=19+2+1  dbl_ovrd=off  reg_retiming=off  block_type=delay
      settings: infoedit=Hardware notes: A delay line is a chain, each link of which is an SRL16 followed by a flip-flop. If register retiming is enabled, the delay line is a chain of flip-flops.  xl_area=[0, 0, 0, 0, 0, 0, 0]  block_version=VER_STRING_GOES_HERE
  Determinant : Subsystem
  Eq Guts : Scope  NumInputPorts=7
      settings: TimeRange=392.7801724137931  YMin=-0.5~-0.25~-1~-1~0~0~0  YMax=0.5~0.15~1~1~1~3500~1  SaveName=ScopeData20
  Phase Corr : Subsystem
  Register{,1..3} (4 alike) : xbsIndex_r4/Register  init=0  rst=off  en=on  dbl_ovrd=off  block_type=register
      Register settings: block_version=VER_STRING_GOES_HERE
      Register1 settings: block_version=VER_STRING_GOES_HERE
      Register2 settings: block_version=8.2.02
      Register3 settings: block_version=8.2.02
  YA*[HBB,-HAB] : Subsystem
  YB*[-HBA,HAA] : Subsystem
  done{1..7} (7 alike) : xbsIndex_r4/Gateway Out  hdl_port=off  timing_constraint=None  locs_specified=off  LOCs={}  block_type=gatewayout
      settings: infoedit="Gateway out block.  Converts Xilinx fixed point inputs into ouputs of type Simulink integer, double, or fixed point.<P><P>Hardware notes:  In hardware these blocks become top level output ports or are discarded, depending on how they are configured."  xl_area=[0 0 0 0 1 0 0]  block_version=VER_STRING_GOES_HERE
  I-eq : Outport  OutDataType=sfix(16)  OutScaling=2^0
  Q-eq : Outport  Port=2  OutDataType=sfix(16)  OutScaling=2^0
  " Xk_index" : Outport  Port=3  OutDataType=sfix(16)  OutScaling=2^0
  Vout : Outport  Port=4  OutDataType=sfix(16)  OutScaling=2^0
  " Sym Index" : Outport  Port=5  OutDataType=sfix(16)  OutScaling=2^0
  tag scope: ChanEstBA_I=global, TMP_scIndex=global
  wires:
  H-I/1 -> Bus Selector/1(H-I)
  H-Q/1 -> Bus Selector/2(H-Q)
  SubCar Index/1 -> Delay20/1, Phase Corr/2(xk_ind), Delay31/1
  Delay6/1 -> Delay7/1
  Vin/1 -> Delay18/1, Phase Corr/1(Vin)
  Delay3/1 -> Delay8/1
  Delay4/1 -> Delay2/1
  Delay7/1 -> Delay12/1
  Delay2/1 -> Delay11/1
  Delay8/1 -> Register1/2, Register/2, Delay10/1
  Sym Index/1 -> Delay19/1, 1LSB1/1, Delay30/1
  Bus Selector/1(AA_I) -> Delay22/1
  Bus Selector/2(AA_Q) -> Delay23/1
  Bus Selector/3(BB_I) -> Delay24/1
  Bus Selector/4(BB_Q) -> Delay25/1
  Bus Selector/5(AB_I) -> Delay26/1
  Bus Selector/6(AB_Q) -> Delay27/1
  Bus Selector/7(BA_I) -> Delay28/1
  Bus Selector/8(BA_Q) -> Delay29/1
  Determinant/1(Re) -> Delay15/1
  Determinant/2(Im) -> Delay16/1
  Y_I/1 -> Bus Selector1/1(Y_I)
  Y_Q/1 -> Bus Selector1/2(Y_Q)
  Bus Selector1/1(YA_I) -> Phase Corr/5(YI_A)
  Bus Selector1/2(YA_Q) -> Phase Corr/6(YQ_A)
  Bus Selector1/3(YB_I) -> Phase Corr/7(YI_B)
  Bus Selector1/4(YB_Q) -> Phase Corr/8(YQ_B)
  YA*[HBB,-HAB]/1(Re) -> Add2/1, done3/1
  YB*[-HBA,HAA]/1(Re) -> Add2/2
  YA*[HBB,-HAB]/2(Im) -> Add1/1, done6/1
  YB*[-HBA,HAA]/2(Im) -> Add1/2
  Delay1/1 -> Delay17/1
  Delay5/1 -> Delay14/1
  Delay9/1 -> Delay13/1, Register2/2, Register3/2
  Complex Divider/1(Re[A/B]) -> Register/1
  Register/1 -> I-eq/1
  Complex Divider/2(Im[A/B]) -> Register1/1
  Register1/1 -> Q-eq/1
  Delay10/1 -> Vout/1
  Delay11/1 -> " Sym Index"/1
  Delay12/1 -> " Xk_index"/1
  1LSB1/1 -> Delay21/1
  Add2/1 -> Register2/1
  Add1/1 -> Register3/1
  Delay14/1 -> Delay4/1
  Delay13/1 -> Delay3/1
  Register2/1 -> Complex Divider/1(Re[A])
  Register3/1 -> Complex Divider/2(Im[A])
  Delay15/1 -> Complex Divider/3(Re[B])
  Delay16/1 -> Complex Divider/4(Im[B])
  Delay17/1 -> Delay6/1
  Phase Corr/1(" YI_A") -> YA*[HBB,-HAB]/1(Re[y]), done4/1
  Phase Corr/2(" YQ_A") -> YA*[HBB,-HAB]/2(Im[y]), done5/1
  Phase Corr/3(" YI_B") -> YB*[-HBA,HAA]/1(Re[y])
  Phase Corr/4(" YQ_B") -> YB*[-HBA,HAA]/2(Im[y])
  Delay18/1 -> Delay9/1
  Delay19/1 -> Delay5/1
  Delay20/1 -> Delay1/1
  Delay21/1 -> YA*[HBB,-HAB]/7(Slot), YB*[-HBA,HAA]/7(Slot)
  Phases I/1 -> Phase Corr/3(Phases I)
  Delay22/1 -> Determinant/1(Re[a]), YB*[-HBA,HAA]/5(Re[h2]), done1/1
  Delay23/1 -> Determinant/2(Im[a]), YB*[-HBA,HAA]/6(Im[h2]), done2/1
  Delay24/1 -> Determinant/3(Re[d]), YA*[HBB,-HAB]/3(Re[h1])
  Delay25/1 -> Determinant/4(Im[d]), YA*[HBB,-HAB]/4(Im[h1])
  Delay26/1 -> Determinant/5(Re[b]), YA*[HBB,-HAB]/5(Re[h2])
  Delay27/1 -> Determinant/6(Im[b]), YA*[HBB,-HAB]/6(Im[h2])
  Delay28/1 -> Determinant/7(Re[c]), YB*[-HBA,HAA]/3(Re[h1]), [ChanEstBA_I]
  Delay29/1 -> Determinant/8(Im[c]), YB*[-HBA,HAA]/4(Im[h1])
  Phases Q/1 -> Phase Corr/4(Phases Q)
  done4/1 -> Eq Guts/1
  done5/1 -> Eq Guts/2
  done1/1 -> Eq Guts/3
  done2/1 -> Eq Guts/4
  done3/1 -> Eq Guts/5
  done6/1 -> Eq Guts/6
  done7/1 -> Eq Guts/7
  Delay31/1 -> Delay33/1
  Delay32/1 -> [TMP_scIndex]
  Delay33/1 -> done7/1, Delay32/1
